simulink library "Renault_Library"  (Simulink 9.2)
settings: Solver=ode3  StartTime=0.0  StopTime=10.0  FixedStep=0.020  RelTol=1e-3  EnableMultiTasking=on
undefined symbols (not defined in the file or in its folder): ABC, AssystemProposal, b, chkval, CoeffA_C, CoeffB_C, CoeffMean_C, ConfTime_C, DiagDelay_C, DividePercent, FailureConfActive_C, FallingEdgeDuration_C, HighLevelThresholdVoltage_C, HighLevelVoltage_C, HighLevelVoltageTunable_C, InitValue_C, InputVoltRangeMax_C, InputVoltRangeMin_C, LowActiveContact_C, LowLevelThresholdVoltage_C, LowLevelVoltage_C, MaxOperatingVoltage_C, MinOperatingVoltage_C, mV2V_conversion, OUT1, OutOfRangeHigh_C, OutOfRangeLow_C, PinSupplySaturationMax, PinSupplySaturationMin, Res1Value_C, Res2Value_C, Res3Value_C, RisingEdgeDuration_C, RSA_CANSignalFailMonitoring, SampleTime_C, service14Table, service19Table, service22Table, service24Table, service28Table, service2ETable, service2FTable, service31Table, service87Table, SizeXStateDID2FTable, SizeXTable14, SizeXTable19, SizeXTable22, SizeXTable24, SizeXTable28, SizeXTable2E, SizeXTable2F, SizeXTable31, SizeXTable87, SizeYStateDID2FTable, SizeYTable14, SizeYTable19, SizeYTable22, SizeYTable24, SizeYTable28 ... (+22)
undefined symbols (not defined in the file): BatteryVoltage_ActivationThreshold_C, FrameStatus_AbsentValue_C, FrameStatus_ConfAbsentValue_C, MemThreshold_C, NAMEOFTHEMODEL_SAMPLE_TIME_CST, NbAbs1_C, NbConfAbs1_C, NbConfPres1_C, NbPres1_C, NOMDUSIGNAL_FaultConfTime_C, NOMDUSIGNAL_FaultRehabTime_C, NOMDUSIGNAL_switchvalue_C, NOMDUSIGNAL_unavailablevalue_C, PeriodFrame1_C, ResetTime_C, WakeUpTime_C
libraries: $bdroot x112, simulink x75
(part 9 of 32)

== system Renault_Library/Complex blocks/Logic & Bit Operations/RSA_CompareToZero ==
  u : Inport
      settings: AttributesFormatString=Type : %<OutDataTypeStr>
  Compare : RelationalOperator  Operator===  OutDataTypeStr=boolean  RndMeth=Nearest  InputSameDT=on
  Constant : Constant  DisableCoverage=on  Value=cons_zero  OutDataTypeStr=Inherit: Inherit via back propagation  SampleTime=-1  PreserveConstantTs=on
  y : Outport
      settings: AttributesFormatString=Type : %<OutDataTypeStr>\nInit :%<InitialOutput>
  wires:
  Constant/1 -> Compare/2
  u/1 -> Compare/1
  Compare/1 -> y/1

== system Renault_Library/Complex blocks/Logic & Bit Operations/RSA_CompareToZero1 ==
  u : Inport
      settings: AttributesFormatString=Type : %<OutDataTypeStr>
  Compare : RelationalOperator  Operator===  OutDataTypeStr=boolean  RndMeth=Nearest  InputSameDT=on
  Constant : Constant  DisableCoverage=on  Value=cons_zero  OutDataTypeStr=Inherit: Inherit via back propagation  SampleTime=-1  PreserveConstantTs=on
  y : Outport
      settings: AttributesFormatString=Type : %<OutDataTypeStr>\nInit :%<InitialOutput>
  wires:
  Constant/1 -> Compare/2
  u/1 -> Compare/1
  Compare/1 -> y/1

== system Renault_Library/Complex blocks/Logic & Bit Operations/RSA_DetectBooleanChange_v01_0 ==
  U : Inport
  Constant1 : Constant  Value=false  OutDataTypeStr=boolean  SampleTime=-1
  Constant2 : Constant  Value=true  OutDataTypeStr=boolean  SampleTime=-1
  Delay_Input1 : UnitDelay  Description=Store in Global RAM  DisableCoverage=on  InitialCondition=false  SampleTime=-1
      settings: AttributesFormatString=IC = %<X0>
  Delay_Input2 : UnitDelay  Description=Store in Global RAM  DisableCoverage=on  InitialCondition=chkini  SampleTime=-1
      settings: AttributesFormatString=IC = %<X0>
  Logical_Operator : Logic  Operator=XOR  OutDataTypeStr=boolean
  Switch : Switch  Criteria=u2 ~= 0  RndMeth=Zero  InputSameDT=on
      settings: AttributesFormatString=Criteria=%<Criteria>
  Y : Outport
  wires:
  Constant1/1 -> Switch/3
  Logical_Operator/1 -> Switch/1
  Switch/1 -> Y/1
  Delay_Input2/1 -> Switch/2
  Constant2/1 -> Delay_Input2/1
  U/1 -> Delay_Input1/1, Logical_Operator/1
  Delay_Input1/1 -> Logical_Operator/2

== system Renault_Library/Complex blocks/Logic & Bit Operations/RSA_DetectChange ==
  note: Edge
  U : Inport
      settings: AttributesFormatString=Type : %<OutDataTypeStr>
  Delay_Input1 : UnitDelay  Description=Store in Global RAM  DisableCoverage=on  InitialCondition=vinit  SampleTime=-1
      settings: AttributesFormatString=IC = %<X0>
  FixPt_Relational_Operator : RelationalOperator  Operator=~=  OutDataTypeStr=boolean  ZeroCross=off  RndMeth=Nearest  InputSameDT=on
  Y : Outport  InitialOutput=0
      settings: AttributesFormatString=Type : %<OutDataTypeStr>\nInit :%<InitialOutput>
  wires:
  FixPt_Relational_Operator/1 -> Y/1
  Delay_Input1/1 -> FixPt_Relational_Operator/2
  U/1 -> FixPt_Relational_Operator/1, Delay_Input1/1

== system Renault_Library/Complex blocks/Logic & Bit Operations/RSA_DetectChange_v01_03 ==
  U : Inport
  Constant1 : Constant  Value=false  OutDataTypeStr=Inherit: Inherit via back propagation  SampleTime=-1
  Constant2 : Constant  Value=false  OutDataTypeStr=boolean  SampleTime=-1
  Delay_Input1 : UnitDelay  Description=Store in Global RAM  DisableCoverage=on  InitialCondition=vinit  SampleTime=-1
      settings: AttributesFormatString=IC = %<X0>
  Delay_Input2 : UnitDelay  Description=Store in Global RAM  DisableCoverage=on  InitialCondition=true  SampleTime=-1
      settings: AttributesFormatString=IC = %<X0>
  FixPt_Relational_Operator : RelationalOperator  Operator=~=  OutDataTypeStr=boolean  ZeroCross=off  RndMeth=Nearest  InputSameDT=on
  Switch : Switch  Criteria=u2 ~= 0  RndMeth=Zero  InputSameDT=on
      settings: AttributesFormatString=Criteria=%<Criteria>
  Y : Outport  InitialOutput=0
  wires:
  Switch/1 -> Y/1  "Edge"
  FixPt_Relational_Operator/1 -> Switch/3
  Constant1/1 -> Switch/1
  Constant2/1 -> Delay_Input2/1
  Delay_Input2/1 -> Switch/2
  Delay_Input1/1 -> FixPt_Relational_Operator/2
  U/1 -> FixPt_Relational_Operator/1, Delay_Input1/1

== system Renault_Library/Complex blocks/Logic & Bit Operations/RSA_DetectChange_v01_04 ==
  U : Inport
  Constant1 : Constant  Value=false  OutDataTypeStr=Inherit: Inherit via back propagation  SampleTime=-1
  Constant2 : Constant  Value=true  OutDataTypeStr=boolean  SampleTime=-1
  Delay_Input1 : UnitDelay  Description=Store in Global RAM  DisableCoverage=on  InitialCondition=vinit  SampleTime=-1
      settings: AttributesFormatString=IC = %<X0>
  Delay_Input2 : UnitDelay  Description=Store in Global RAM  DisableCoverage=on  InitialCondition=chkval  SampleTime=-1
      settings: AttributesFormatString=IC = %<X0>
  FixPt_Relational_Operator : RelationalOperator  Operator=~=  OutDataTypeStr=boolean  ZeroCross=off  RndMeth=Nearest  InputSameDT=on
  Switch : Switch  Criteria=u2 ~= 0  RndMeth=Zero  InputSameDT=on
      settings: AttributesFormatString=Criteria=%<Criteria>
  Y : Outport  InitialOutput=0
  wires:
  U/1 -> Delay_Input1/1, FixPt_Relational_Operator/1
  Delay_Input1/1 -> FixPt_Relational_Operator/2
  Switch/1 -> Y/1  "Edge"
  Constant2/1 -> Delay_Input2/1
  FixPt_Relational_Operator/1 -> Switch/1
  Constant1/1 -> Switch/3
  Delay_Input2/1 -> Switch/2

== system Renault_Library/Complex blocks/Logic & Bit Operations/RSA_DetectChange_v01_05 ==
  U : Inport
  Add : Sum  Inputs=+-  OutDataTypeStr=Inherit: Inherit via back propagation  RndMeth=Zero  InputSameDT=on
      settings: AttributesFormatString=SAT = %<SaturateOnIntegerOverflow>
  Add1 : Sum  OutDataTypeStr=Inherit: Inherit via back propagation  RndMeth=Zero  InputSameDT=on
      settings: AttributesFormatString=SAT = %<SaturateOnIntegerOverflow>
  Con3 : Constant  Value=tol  OutDataTypeStr=Inherit: Inherit via back propagation
  Con5 : Constant  Value=tol  OutDataTypeStr=Inherit: Inherit via back propagation
  Constant2 : Constant  Value=true  OutDataTypeStr=boolean  SampleTime=-1
  Constant3 : Constant  Value=false  OutDataTypeStr=boolean  SampleTime=-1
  Delay_Input1 : UnitDelay  Description=Store in Global RAM  DisableCoverage=on  InitialCondition=vinit  SampleTime=-1
      settings: AttributesFormatString=IC = %<X0>
  Delay_Input2 : UnitDelay  Description=Store in Global RAM  DisableCoverage=on  InitialCondition=false  SampleTime=-1
      settings: AttributesFormatString=IC = %<X0>
  Logical_Operator12 : Logic  Operator=XOR  OutDataTypeStr=boolean
  Switch : Switch  Criteria=u2 ~= 0  RndMeth=Zero  InputSameDT=on
      settings: AttributesFormatString=Criteria=%<Criteria>
  Terminator : Terminator
  Terminator1 : Terminator
  Var_RelationalOper1 : RelationalOperator  Operator=>  OutDataTypeStr=boolean  RndMeth=Nearest  InputSameDT=on
  Var_RelationalOper2 : RelationalOperator  Operator=<  OutDataTypeStr=boolean  RndMeth=Nearest  InputSameDT=on
  Y : Outport  InitialOutput=0
  wires:
  Delay_Input2/1 -> Switch/2
  [fromRelOpe] -> Switch/3
  Constant2/1 -> Delay_Input2/1
  Switch/1 -> Y/1  "Edge"
  Delay_Input1/1 -> [Uprev]
  Constant3/1 -> [vchk_val]
  [compare_out] -> [fromRelOpe], Switch/1
  [vchk_val] -> Terminator/1
  [fromRelOpe] -> Terminator1/1
  Logical_Operator12/1 -> [compare_out]
  Con3/1 -> Add/2
  Con5/1 -> Add1/2
  Add/1 -> Var_RelationalOper1/2
  Var_RelationalOper1/1 -> Logical_Operator12/1
  Var_RelationalOper2/1 -> Logical_Operator12/2
  [U] -> Var_RelationalOper1/1
  [U] -> Var_RelationalOper2/1
  Add1/1 -> Var_RelationalOper2/2
  [Uprev] -> Add/1
  [Uprev] -> Add1/1
  U/1 -> [U], Delay_Input1/1

== system Renault_Library/Complex blocks/Logic & Bit Operations/RSA_DetectDecrease ==
  note: Edge
  U : Inport
      settings: AttributesFormatString=Type : %<OutDataTypeStr>
  Delay_Input1 : UnitDelay  Description=Store in Global RAM  DisableCoverage=on  InitialCondition=vinit  SampleTime=-1
      settings: AttributesFormatString=IC = %<X0>
  FixPt_Relational_Operator : RelationalOperator  Operator=<  OutDataTypeStr=boolean  ZeroCross=off  RndMeth=Nearest  InputSameDT=on
  Y : Outport  InitialOutput=0
      settings: AttributesFormatString=Type : %<OutDataTypeStr>\nInit :%<InitialOutput>
  wires:
  U/1 -> Delay_Input1/1, FixPt_Relational_Operator/1
  Delay_Input1/1 -> FixPt_Relational_Operator/2
  FixPt_Relational_Operator/1 -> Y/1

== system Renault_Library/Complex blocks/Logic & Bit Operations/RSA_DetectDecrease_v01_03 ==
  U : Inport
  Constant1 : Constant  Value=false  OutDataTypeStr=Inherit: Inherit via back propagation  SampleTime=-1
  Constant2 : Constant  Value=false  OutDataTypeStr=boolean  SampleTime=-1
  Delay_Input1 : UnitDelay  Description=Store in Global RAM  DisableCoverage=on  InitialCondition=vinit  SampleTime=-1
      settings: AttributesFormatString=IC = %<X0>
  Delay_Input2 : UnitDelay  Description=Store in Global RAM  DisableCoverage=on  InitialCondition=true  SampleTime=-1
      settings: AttributesFormatString=IC = %<X0>
  FixPt_Relational_Operator : RelationalOperator  Operator=<  OutDataTypeStr=boolean  ZeroCross=off  RndMeth=Nearest  InputSameDT=on
  Switch : Switch  Criteria=u2 ~= 0  RndMeth=Zero  InputSameDT=on
      settings: AttributesFormatString=Criteria=%<Criteria>
  Y : Outport  InitialOutput=0
  wires:
  Delay_Input1/1 -> FixPt_Relational_Operator/2
  U/1 -> FixPt_Relational_Operator/1, Delay_Input1/1
  Delay_Input2/1 -> Switch/2
  Constant2/1 -> Delay_Input2/1
  Constant1/1 -> Switch/1
  FixPt_Relational_Operator/1 -> Switch/3
  Switch/1 -> Y/1  "Edge"

== system Renault_Library/Complex blocks/Logic & Bit Operations/RSA_DetectDecrease_v01_04 ==
  U : Inport
  Constant1 : Constant  Value=false  OutDataTypeStr=Inherit: Inherit via back propagation  SampleTime=-1
  Constant2 : Constant  Value=true  OutDataTypeStr=boolean  SampleTime=-1
  Delay_Input1 : UnitDelay  Description=Store in Global RAM  DisableCoverage=on  InitialCondition=vinit  SampleTime=-1
      settings: AttributesFormatString=IC = %<X0>
  Delay_Input2 : UnitDelay  Description=Store in Global RAM  DisableCoverage=on  InitialCondition=chkval  SampleTime=-1
      settings: AttributesFormatString=IC = %<X0>
  FixPt_Relational_Operator : RelationalOperator  Operator=<  OutDataTypeStr=boolean  ZeroCross=off  RndMeth=Nearest  InputSameDT=on
  Switch : Switch  Criteria=u2 ~= 0  RndMeth=Zero  InputSameDT=on
      settings: AttributesFormatString=Criteria=%<Criteria>
  Y : Outport  InitialOutput=0
  wires:
  Delay_Input1/1 -> FixPt_Relational_Operator/2
  U/1 -> FixPt_Relational_Operator/1, Delay_Input1/1
  Delay_Input2/1 -> Switch/2
  Constant2/1 -> Delay_Input2/1
  Switch/1 -> Y/1  "Edge"
  FixPt_Relational_Operator/1 -> Switch/1
  Constant1/1 -> Switch/3

== system Renault_Library/Complex blocks/Logic & Bit Operations/RSA_DetectIncDec_V01_00 ==
  U : Inport
  Constant2 : Constant  Value=true  OutDataTypeStr=boolean  SampleTime=-1
  Constant3 : Constant  Value=false  OutDataTypeStr=boolean  SampleTime=-1
  Delay_Input1 : UnitDelay  Description=Store in Global RAM  DisableCoverage=on  InitialCondition=vinit  SampleTime=-1
      settings: AttributesFormatString=IC = %<X0>
  Delay_Input2 : UnitDelay  Description=Store in Global RAM  DisableCoverage=on  InitialCondition=false  SampleTime=-1
      settings: AttributesFormatString=IC = %<X0>
  FixPt_Relational_Operator : RelationalOperator  Operator=<  OutDataTypeStr=boolean  ZeroCross=off  RndMeth=Nearest  InputSameDT=on
  Switch : Switch  Criteria=u2 ~= 0  RndMeth=Zero  InputSameDT=on
      settings: AttributesFormatString=Criteria=%<Criteria>
  Terminator : Terminator
  Terminator1 : Terminator
  Y : Outport  InitialOutput=0
  wires:
  Switch/1 -> Y/1  "Edge"
  [fromRelOpe] -> Terminator1/1
  [vchk_val] -> Terminator/1
  FixPt_Relational_Operator/1 -> Switch/1, [fromRelOpe]
  [vchk_val] -> Switch/3
  Constant2/1 -> Delay_Input2/1
  Constant3/1 -> [vchk_val]
  Delay_Input2/1 -> Switch/2
  U/1 -> Delay_Input1/1, FixPt_Relational_Operator/1
  Delay_Input1/1 -> FixPt_Relational_Operator/2

== system Renault_Library/Complex blocks/Logic & Bit Operations/RSA_DetectIncrease ==
  note: Edge
  U : Inport
      settings: AttributesFormatString=Type : %<OutDataTypeStr>
  Delay_Input1 : UnitDelay  Description=Store in Global RAM  DisableCoverage=on  InitialCondition=vinit  SampleTime=-1
      settings: AttributesFormatString=IC = %<X0>
  FixPt_Relational_Operator : RelationalOperator  Operator=>  OutDataTypeStr=boolean  ZeroCross=off  RndMeth=Nearest  InputSameDT=on
  Y : Outport  InitialOutput=0
      settings: AttributesFormatString=Type : %<OutDataTypeStr>\nInit :%<InitialOutput>
  wires:
  U/1 -> Delay_Input1/1, FixPt_Relational_Operator/1
  Delay_Input1/1 -> FixPt_Relational_Operator/2
  FixPt_Relational_Operator/1 -> Y/1

== system Renault_Library/Complex blocks/Logic & Bit Operations/RSA_DetectIncrease_v01_03 ==
  U : Inport
  Constant1 : Constant  Value=false  OutDataTypeStr=Inherit: Inherit via back propagation  SampleTime=-1
  Constant2 : Constant  Value=false  OutDataTypeStr=boolean  SampleTime=-1
  Delay_Input1 : UnitDelay  Description=Store in Global RAM  DisableCoverage=on  InitialCondition=vinit  SampleTime=-1
      settings: AttributesFormatString=IC = %<X0>
  Delay_Input2 : UnitDelay  Description=Store in Global RAM  DisableCoverage=on  InitialCondition=true  SampleTime=-1
      settings: AttributesFormatString=IC = %<X0>
  FixPt_Relational_Operator : RelationalOperator  Operator=>  OutDataTypeStr=boolean  ZeroCross=off  RndMeth=Nearest  InputSameDT=on
  Switch : Switch  Criteria=u2 ~= 0  RndMeth=Zero  InputSameDT=on
      settings: AttributesFormatString=Criteria=%<Criteria>
  Y : Outport  InitialOutput=0
  wires:
  Delay_Input1/1 -> FixPt_Relational_Operator/2
  U/1 -> FixPt_Relational_Operator/1, Delay_Input1/1
  Delay_Input2/1 -> Switch/2
  Constant2/1 -> Delay_Input2/1
  Constant1/1 -> Switch/1
  Switch/1 -> Y/1  "Edge"
  FixPt_Relational_Operator/1 -> Switch/3

== system Renault_Library/Complex blocks/Logic & Bit Operations/RSA_DetectIncrease_v01_04 ==
  U : Inport
  Constant1 : Constant  Value=false  OutDataTypeStr=Inherit: Inherit via back propagation  SampleTime=-1
  Constant2 : Constant  Value=true  OutDataTypeStr=boolean  SampleTime=-1
  Delay_Input1 : UnitDelay  Description=Store in Global RAM  DisableCoverage=on  InitialCondition=vinit  SampleTime=-1
      settings: AttributesFormatString=IC = %<X0>
  Delay_Input2 : UnitDelay  Description=Store in Global RAM  DisableCoverage=on  InitialCondition=chkval  SampleTime=-1
      settings: AttributesFormatString=IC = %<X0>
  FixPt_Relational_Operator : RelationalOperator  Operator=>  OutDataTypeStr=boolean  ZeroCross=off  RndMeth=Nearest  InputSameDT=on
  Switch : Switch  Criteria=u2 ~= 0  RndMeth=Zero  InputSameDT=on
      settings: AttributesFormatString=Criteria=%<Criteria>
  Y : Outport  InitialOutput=0
  wires:
  Switch/1 -> Y/1  "Edge"
  Constant2/1 -> Delay_Input2/1
  Delay_Input2/1 -> Switch/2
  U/1 -> Delay_Input1/1, FixPt_Relational_Operator/1
  Delay_Input1/1 -> FixPt_Relational_Operator/2
  FixPt_Relational_Operator/1 -> Switch/1
  Constant1/1 -> Switch/3

== system Renault_Library/Complex blocks/Logic & Bit Operations/RSA_EdgeDetect ==
  In : Inport
      settings: AttributesFormatString=Type : %<OutDataType>\nScaling : %<OutScaling>
  IC : Inport  Port=2
      settings: AttributesFormatString=Type : %<OutDataType>\nScaling : %<OutScaling>
  Edge_type : Constant  Value=Edge_type  OutDataTypeStr=uint8  SampleTime=-1
  Logical_Operator : Logic  OutDataTypeStr=boolean
  Logical_Operator1 : Logic  Operator=NOT  OutDataTypeStr=boolean
  Logical_Operator2 : Logic  OutDataTypeStr=boolean
  Logical_Operator3 : Logic  Operator=NOT  OutDataTypeStr=boolean
  Logical_Operator4 : Logic  Operator=XOR  OutDataTypeStr=boolean
  Multiport_Switch : MultiPortSwitch  RndMeth=Zero  SaturateOnIntegerOverflow=on  InputSameDT=on
  RSA_UnitDelayExternalIC_v01_03 : $bdroot/Complex blocks/Discrete/RSA_UnitDelayExternalIC_v01_03  Tag=[LIB]  SystemSampleTime=-1
      settings: AttributesFormatString=%<MaskType> : Ver : 01.03
  Out : Outport
      settings: AttributesFormatString=Type : %<OutDataType>\nScaling : %<OutScaling>\nInit :%<InitialOutput>
  wires:
  In/1 -> Logical_Operator/1, Logical_Operator3/1, Logical_Operator4/1, RSA_UnitDelayExternalIC_v01_03/1
  Logical_Operator1/1 -> Logical_Operator/2
  Logical_Operator3/1 -> Logical_Operator2/1
  Logical_Operator/1 -> Multiport_Switch/2  "Rising"
  Logical_Operator2/1 -> Multiport_Switch/3  "Falling"
  Logical_Operator4/1 -> Multiport_Switch/4  "Either"
  Edge_type/1 -> Multiport_Switch/1
  Multiport_Switch/1 -> Out/1
  IC/1 -> RSA_UnitDelayExternalIC_v01_03/2
  RSA_UnitDelayExternalIC_v01_03/1 -> [y]
  [y] -> Logical_Operator1/1
  [y] -> Logical_Operator2/2
  [y] -> Logical_Operator4/2

== system Renault_Library/Complex blocks/Logic & Bit Operations/RSA_EdgeDetect_V01_02 ==
  In : Inport
      settings: AttributesFormatString=Type : %<OutDataType>\nScaling : %<OutScaling>
  IC : Inport  Port=2
      settings: AttributesFormatString=Type : %<OutDataType>\nScaling : %<OutScaling>
  Logical_Operator : Logic  OutDataTypeStr=boolean
  Logical_Operator1 : Logic  Operator=NOT  OutDataTypeStr=boolean
  Logical_Operator2 : Logic  OutDataTypeStr=boolean
  Logical_Operator3 : Logic  Operator=NOT  OutDataTypeStr=boolean
  Logical_Operator4 : Logic  Operator=XOR  OutDataTypeStr=boolean
  RSA_UnitDelayExternalIC_v01_03 : $bdroot/Complex blocks/Discrete/RSA_UnitDelayExternalIC_v01_03  Tag=[LIB]  SystemSampleTime=-1
      settings: AttributesFormatString=%<MaskType> : Ver : 01.03
  Terminator : Terminator
  Terminator1 : Terminator
  Terminator2 : Terminator
  Out : Outport
      settings: AttributesFormatString=Type : %<OutDataType>\nScaling : %<OutScaling>\nInit :%<InitialOutput>
  wires:
  In/1 -> Logical_Operator/1, Logical_Operator3/1, Logical_Operator4/1, RSA_UnitDelayExternalIC_v01_03/1
  Logical_Operator1/1 -> Logical_Operator/2
  Logical_Operator3/1 -> Logical_Operator2/1
  IC/1 -> RSA_UnitDelayExternalIC_v01_03/2
  RSA_UnitDelayExternalIC_v01_03/1 -> [y]
  [y] -> Logical_Operator1/1
  [y] -> Logical_Operator2/2
  [y] -> Logical_Operator4/2
  [FallingEdge] -> Out/1
  Logical_Operator/1 -> [RisingEdge]  "Rising"
  Logical_Operator2/1 -> [FallingEdge]  "Falling"
  Logical_Operator4/1 -> [Either]  "Either"
  [RisingEdge] -> Terminator/1
  [FallingEdge] -> Terminator1/1
  [Either] -> Terminator2/1

== system Renault_Library/Complex blocks/Lookup Tables/RSA_Cosine_v01_03 ==
  u : Inport
      settings: AttributesFormatString=Type : %<OutDataTypeStr>
  Amp25 : Sum  Inputs=+-  OutDataTypeStr=Inherit: Inherit via back propagation  InputSameDT=on  LockScale=on
      settings: AttributesFormatString=SAT = %<SaturateOnIntegerOverflow>
  Amp75 : Sum  Inputs=+-  OutDataTypeStr=Inherit: Inherit via back propagation  InputSameDT=on  LockScale=on
      settings: AttributesFormatString=SAT = %<SaturateOnIntegerOverflow>
  Constant11 : Constant  Value=NVAL  OutDataTypeStr=Inherit: Inherit via back propagation  SampleTime=-1
  DataTypeConversion : DataTypeConversion  OutDataTypeStr=fixdt(0, OutputWordLength, OutputWordLength)
  GTEp75 : RelationalOperator  OutDataTypeStr=boolean  ZeroCross=off  RndMeth=Nearest  InputSameDT=on
  LTEp25 : RelationalOperator  Operator=<=  OutDataTypeStr=boolean  ZeroCross=off  RndMeth=Nearest  InputSameDT=on
  LTEp50 : RelationalOperator  Operator=<=  OutDataTypeStr=boolean  ZeroCross=off  RndMeth=Nearest  InputSameDT=on
  Look_Up_Table : Lookup  InputValues=k  Table=b  LookUpMeth=Interpolation-Use End Values  OutDataTypeStr=fixdt(1, OutputWordLength, OutputFracLength)  RndMeth=Zero  SaturateOnIntegerOverflow=on
  Point25 : Constant  Value=0.25  OutDataTypeStr=fixdt(0, OutputWordLength, OutputWordLength)
  Point5 : Constant  Value=0.5  OutDataTypeStr=fixdt(0, OutputWordLength, OutputWordLength)
  Point75 : Constant  Value=0.75  OutDataTypeStr=fixdt(0, OutputWordLength, OutputWordLength)
  Product2 : Product  OutDataTypeStr=Inherit: Inherit via back propagation  RndMeth=Zero
      settings: AttributesFormatString=SAT = %<SaturateOnIntegerOverflow>
  QuadHandle1a : Switch  Criteria=u2 ~= 0  ZeroCross=off  InputSameDT=on
      settings: AttributesFormatString=Criteria=%<Criteria>
  QuadHandle1b : Switch  Criteria=u2 ~= 0  ZeroCross=off  InputSameDT=on
      settings: AttributesFormatString=Criteria=%<Criteria>
  QuadHandle2 : Switch  Criteria=u2 ~= 0  ZeroCross=off  InputSameDT=on
      settings: AttributesFormatString=Criteria=%<Criteria>
  SignCorrected : Switch  Criteria=u2 ~= 0  ZeroCross=off  InputSameDT=on
      settings: AttributesFormatString=Criteria=%<Criteria>
  firstor4thQuad : Logic  Operator=OR  OutDataTypeStr=boolean
  p25mA : Sum  Inputs=+-  OutDataTypeStr=Inherit: Inherit via back propagation  InputSameDT=on  LockScale=on
      settings: AttributesFormatString=SAT = %<SaturateOnIntegerOverflow>
  p75mA : Sum  Inputs=+-  OutDataTypeStr=Inherit: Inherit via back propagation  InputSameDT=on  LockScale=on
      settings: AttributesFormatString=SAT = %<SaturateOnIntegerOverflow>
  y : Outport
      settings: AttributesFormatString=Type : %<OutDataTypeStr>\nInit :%<InitialOutput>
  wires:
  QuadHandle2/1 -> Look_Up_Table/1
  Constant11/1 -> Product2/2
  SignCorrected/1 -> y/1
  Product2/1 -> SignCorrected/3
  Look_Up_Table/1 -> Product2/1, SignCorrected/1
  [fourthquad] -> firstor4thQuad/2
  [firstquad] -> firstor4thQuad/1
  firstor4thQuad/1 -> SignCorrected/2
  [Point75] -> p75mA/1
  QuadHandle1b/1 -> QuadHandle2/3
  QuadHandle1a/1 -> QuadHandle2/1
  LTEp50/1 -> QuadHandle2/2
  Point5/1 -> LTEp50/2
  p75mA/1 -> QuadHandle1b/3
  [Point75] -> Amp75/2
  [Point75] -> GTEp75/2
  Point75/1 -> [Point75]
  Amp75/1 -> QuadHandle1b/1
  GTEp75/1 -> [fourthquad], QuadHandle1b/2
  [Point25] -> p25mA/1
  [Point25] -> Amp25/2
  [Point25] -> LTEp25/2
  Point25/1 -> [Point25]
  Amp25/1 -> QuadHandle1a/3
  p25mA/1 -> QuadHandle1a/1
  LTEp25/1 -> [firstquad], QuadHandle1a/2
  DataTypeConversion/1 -> LTEp50/1, p75mA/2, GTEp75/1, Amp75/1, Amp25/1, p25mA/2, LTEp25/1
  u/1 -> DataTypeConversion/1

== system Renault_Library/Complex blocks/Lookup Tables/RSA_Cosine_v01_04 ==
  u : Inport
      settings: AttributesFormatString=Type : %<OutDataTypeStr>
  Amp25 : Sum  Inputs=+-  OutDataTypeStr=Inherit: Inherit via back propagation  RndMeth=Zero  InputSameDT=on  LockScale=on
      settings: AttributesFormatString=SAT = %<SaturateOnIntegerOverflow>
  Amp75 : Sum  Inputs=+-  OutDataTypeStr=Inherit: Inherit via back propagation  RndMeth=Zero  InputSameDT=on  LockScale=on
      settings: AttributesFormatString=SAT = %<SaturateOnIntegerOverflow>
  Constant11 : Constant  Value=CONS_M1  OutDataTypeStr=Inherit: Inherit via back propagation  SampleTime=-1
  DataTypeConversion : DataTypeConversion  OutDataTypeStr=fixdt(0, OutputWordLength, OutputWordLength)  RndMeth=Zero
  GTEp75 : RelationalOperator  OutDataTypeStr=boolean  ZeroCross=off  RndMeth=Nearest  InputSameDT=on
  LTEp25 : RelationalOperator  Operator=<=  OutDataTypeStr=boolean  ZeroCross=off  RndMeth=Nearest  InputSameDT=on
  LTEp50 : RelationalOperator  Operator=<=  OutDataTypeStr=boolean  ZeroCross=off  RndMeth=Nearest  InputSameDT=on
  Look_Up_Table : Lookup  InputValues=k  Table=b  LookUpMeth=Interpolation-Use End Values  OutDataTypeStr=fixdt(1, OutputWordLength, OutputFracLength)  RndMeth=Zero  SaturateOnIntegerOverflow=on
  Point25 : Constant  Value=CONS_P25  OutDataTypeStr=fixdt(0, OutputWordLength, OutputWordLength)
  Point5 : Constant  Value=CONS_P5  OutDataTypeStr=fixdt(0, OutputWordLength, OutputWordLength)
  Point75 : Constant  Value=CONS_P75  OutDataTypeStr=fixdt(0, OutputWordLength, OutputWordLength)
  Product2 : Product  OutDataTypeStr=Inherit: Inherit via back propagation  RndMeth=Zero
      settings: AttributesFormatString=SAT = %<SaturateOnIntegerOverflow>
  QuadHandle1a : Switch  Criteria=u2 ~= 0  RndMeth=Zero  ZeroCross=off  InputSameDT=on
      settings: AttributesFormatString=Criteria=%<Criteria>
  QuadHandle1b : Switch  Criteria=u2 ~= 0  RndMeth=Zero  ZeroCross=off  InputSameDT=on
      settings: AttributesFormatString=Criteria=%<Criteria>
  QuadHandle2 : Switch  Criteria=u2 ~= 0  RndMeth=Zero  ZeroCross=off  InputSameDT=on
      settings: AttributesFormatString=Criteria=%<Criteria>
  SignCorrected : Switch  Criteria=u2 ~= 0  RndMeth=Zero  ZeroCross=off  InputSameDT=on
      settings: AttributesFormatString=Criteria=%<Criteria>
  firstor4thQuad : Logic  Operator=OR  OutDataTypeStr=boolean
  p25mA : Sum  Inputs=+-  OutDataTypeStr=Inherit: Inherit via back propagation  RndMeth=Zero  InputSameDT=on  LockScale=on
      settings: AttributesFormatString=SAT = %<SaturateOnIntegerOverflow>
  p75mA : Sum  Inputs=+-  OutDataTypeStr=Inherit: Inherit via back propagation  RndMeth=Zero  InputSameDT=on  LockScale=on
      settings: AttributesFormatString=SAT = %<SaturateOnIntegerOverflow>
  y : Outport
      settings: AttributesFormatString=Type : %<OutDataTypeStr>\nInit :%<InitialOutput>
  wires:
  QuadHandle2/1 -> Look_Up_Table/1
  Constant11/1 -> Product2/2
  SignCorrected/1 -> y/1
  Product2/1 -> SignCorrected/3
  Look_Up_Table/1 -> Product2/1, SignCorrected/1
  [fourthquad] -> firstor4thQuad/2
  [firstquad] -> firstor4thQuad/1
  firstor4thQuad/1 -> SignCorrected/2
  [Point75] -> p75mA/1
  QuadHandle1b/1 -> QuadHandle2/3
  QuadHandle1a/1 -> QuadHandle2/1
  LTEp50/1 -> QuadHandle2/2
  Point5/1 -> LTEp50/2
  p75mA/1 -> QuadHandle1b/3
  [Point75] -> Amp75/2
  [Point75] -> GTEp75/2
  Point75/1 -> [Point75]
  Amp75/1 -> QuadHandle1b/1
  GTEp75/1 -> [fourthquad], QuadHandle1b/2
  [Point25] -> p25mA/1
  [Point25] -> Amp25/2
  [Point25] -> LTEp25/2
  Point25/1 -> [Point25]
  Amp25/1 -> QuadHandle1a/3
  p25mA/1 -> QuadHandle1a/1
  LTEp25/1 -> [firstquad], QuadHandle1a/2
  DataTypeConversion/1 -> LTEp50/1, p75mA/2, GTEp75/1, Amp75/1, Amp25/1, p25mA/2, LTEp25/1
  u/1 -> DataTypeConversion/1

== system Renault_Library/Complex blocks/Lookup Tables/RSA_LookupTableDynamic_v02_00 ==
  x : Inport
  xdat : Inport  Port=2
  ydat : Inport  Port=3
  Constant4 : Constant  Value=cons1  OutDataTypeStr=uint8  SampleTime=-1
  DataTypeConversion1 : DataTypeConversion  OutDataTypeStr=uint8  RndMeth=Zero
  DataTypeConversion1_16 : DataTypeConversion  OutDataTypeStr=uint8  RndMeth=Zero
  Demux : Demux  Outputs=2
  Demux1 : Demux  Outputs=2
  Divide : Product  Tag=[LIB]  Inputs=*/  OutDataTypeStr=datt  SaturateOnIntegerOverflow=on  RndMeth=Zero
      settings: AttributesFormatString=SAT = %<SaturateOnIntegerOverflow>
  MinMax : MinMax  OutDataTypeStr=Inherit: Inherit via back propagation  RndMeth=Zero  InputSameDT=on
  MinMax1 : MinMax  Function=max  OutDataTypeStr=Inherit: Inherit via back propagation  RndMeth=Zero  InputSameDT=on
  Mux2 : Mux  Inputs=2
  Mux3 : Mux  Inputs=2
  Mux4 : Mux  Inputs=2
  Product1 : Product  OutDataTypeStr=Inherit: Inherit via back propagation  RndMeth=Nearest  SaturateOnIntegerOverflow=on
      settings: AttributesFormatString=SAT = %<SaturateOnIntegerOverflow>
  Product2 : Product  OutDataTypeStr=Inherit: Inherit via back propagation  RndMeth=Nearest  SaturateOnIntegerOverflow=on
      settings: AttributesFormatString=SAT = %<SaturateOnIntegerOverflow>
  RelationalOperator : RelationalOperator  Tag=AM,Std,2,1;CMP,Operator;  Operator=~=  OutDataTypeStr=boolean  RndMeth=Nearest  InputSameDT=on
  RelationalOperator1 : RelationalOperator  OutDataTypeStr=boolean  RndMeth=Nearest  InputSameDT=on
  RelationalOperator2 : RelationalOperator  Operator=<=  OutDataTypeStr=boolean  RndMeth=Nearest  InputSameDT=on
  RelationalOperator3 : RelationalOperator  OutDataTypeStr=boolean  RndMeth=Nearest  InputSameDT=on
  Relational_Operator : RelationalOperator  Operator=<=  OutDataTypeStr=boolean  RndMeth=Nearest  InputSameDT=on
  Relational_Operator1 : RelationalOperator  OutDataTypeStr=boolean  RndMeth=Nearest  InputSameDT=on
  Relational_Operator2 : RelationalOperator  Operator===  OutDataTypeStr=boolean  RndMeth=Nearest  InputSameDT=on
  Relational_Operator3 : RelationalOperator  Operator=>  OutDataTypeStr=boolean  RndMeth=Nearest  InputSameDT=on
  Selector{1,3..5} (4 alike) : Selector  IndexOptions=Index vector (port)  Indices=SelIdx  OutputSizes=1
  Sum1 : Sum  Inputs=+-  OutDataTypeStr=Inherit: Inherit via back propagation  RndMeth=Zero  SaturateOnIntegerOverflow=on  InputSameDT=on
      settings: AttributesFormatString=SAT = %<SaturateOnIntegerOverflow>
  Sum{2,6..8} (4 alike) : Sum  Inputs=-+  OutDataTypeStr=Inherit: Inherit via back propagation  RndMeth=Zero  SaturateOnIntegerOverflow=on  InputSameDT=on
      settings: AttributesFormatString=SAT = %<SaturateOnIntegerOverflow>
  Sum3 : Sum  OutDataTypeStr=Inherit: Inherit via back propagation  RndMeth=Zero  SaturateOnIntegerOverflow=on  InputSameDT=on
      settings: AttributesFormatString=SAT = %<SaturateOnIntegerOverflow>
  Sum4 : Sum  OutDataTypeStr=Inherit: Inherit via back propagation  RndMeth=Zero  SaturateOnIntegerOverflow=on  InputSameDT=on
      settings: AttributesFormatString=SAT = %<SaturateOnIntegerOverflow>
  Sum_of_Elements : Sum  Inputs=+  OutDataTypeStr=Inherit: Inherit via back propagation  RndMeth=Zero  InputSameDT=on
      settings: AttributesFormatString=SAT = %<SaturateOnIntegerOverflow>
  SumofElements2 : Sum  Inputs=+  OutDataTypeStr=Inherit: Inherit via back propagation  RndMeth=Zero  InputSameDT=on
      settings: AttributesFormatString=SAT = %<SaturateOnIntegerOverflow>
  Switch{,1..4} (5 alike) : Switch  Criteria=u2 ~= 0  RndMeth=Zero  InputSameDT=on
      settings: AttributesFormatString=Criteria=%<Criteria>
  Switch5 : Switch  Tag=GEN,Threshold;AM,Switch,2,1;CMP,Threshold;  Criteria=u2 ~= 0  RndMeth=Zero  SaturateOnIntegerOverflow=on  InputSameDT=on
      settings: AttributesFormatString=Criteria=%<Criteria>
  imm_zero : Constant  Tag=AM,Constant,0,1,Value;CMP,Value;  Value=divchk  OutDataTypeStr=Inherit: Inherit via back propagation  SampleTime=-1
  tempx1 : Selector  IndexOptions=Index vector (port)  Indices=SelIdx  OutputSizes=2
  tempx2 : Selector  IndexOptions=Index vector (port)  Indices=SelIdx  OutputSizes=2
  y : Outport
  wires:
  [temp2] -> tempx2/2
  [cons1] -> Mux2/1
  Switch2/1 -> [temp2], tempx1/2
  [cons1] -> Mux2/2
  Switch4/1 -> Switch2/3
  Mux4/1 -> Switch4/1
  [ylen] -> Mux4/2
  [ylen] -> Mux4/1
  RelationalOperator3/1 -> Switch4/2
  [ylen] -> Selector3/2
  Selector3/1 -> RelationalOperator3/2
  [x] -> RelationalOperator3/1
  [xdat] -> Selector3/1
  Mux2/1 -> Switch2/1
  Mux3/1 -> Switch4/3
  [xdat] -> RelationalOperator1/2
  [x] -> RelationalOperator1/1
  RelationalOperator2/1 -> Switch2/2
  Selector1/1 -> RelationalOperator2/2
  [x] -> RelationalOperator2/1
  [xdat] -> Selector1/1
  [cons1] -> Selector1/2
  SumofElements2/1 -> Mux3/1, Sum4/1
  [cons1] -> Sum4/2
  Sum4/1 -> Mux3/2
  DataTypeConversion1/1 -> SumofElements2/1
  RelationalOperator1/1 -> DataTypeConversion1/1
  Switch1/1 -> Switch/3
  Relational_Operator1/1 -> Switch1/2
  [x] -> Relational_Operator1/1
  Relational_Operator/1 -> Switch/2
  [x] -> Relational_Operator/1
  [xdat] -> MinMax/1
  [xdat] -> MinMax1/1
  MinMax1/1 -> Relational_Operator1/2
  MinMax/1 -> Relational_Operator/2
  Selector4/1 -> Switch/1
  [ydat] -> Selector4/1
  Selector5/1 -> Switch1/1
  [ydat] -> Selector5/1, Relational_Operator2/1, Relational_Operator2/2
  DataTypeConversion1_16/1 -> Sum_of_Elements/1
  Relational_Operator2/1 -> DataTypeConversion1_16/1
  Sum_of_Elements/1 -> [ylen], Selector5/2
  Demux/1 -> Sum1/2, Sum6/1
  xdat/1 -> tempx1/1, [xdat]
  Demux/2 -> Sum6/2
  x/1 -> Sum1/1, [x]
  ydat/1 -> [ydat], tempx2/1
  tempx2/1 -> Demux1/1
  Switch/1 -> y/1
  tempx1/1 -> Demux/1
  [cons1] -> Selector4/2
  Constant4/1 -> [cons1]
  [ydat2] -> Sum7/1
  [ydat1] -> Sum7/2
  [ydat1] -> Sum2/1
  [ydat2] -> Sum2/2
  [ydat1] -> Sum8/2
  Sum8/1 -> Switch3/1
  Sum3/1 -> Switch3/3
  [ydat2] -> Relational_Operator3/2
  [ydat1] -> Relational_Operator3/1
  [ydat1] -> Sum3/1
  Product1/1 -> Sum8/1  "y0"
  Sum7/1 -> Product1/1
  Product2/1 -> Sum3/2
  Sum2/1 -> Product2/2
  Relational_Operator3/1 -> Switch3/2
  Demux1/1 -> [ydat1]
  Demux1/2 -> [ydat2]
  Switch3/1 -> Switch1/3
  Switch5/1 -> Product2/1, Product1/2
  Sum1/1 -> [nume]
  Sum6/1 -> [dnom]
  imm_zero/1 -> RelationalOperator/2
  Divide/1 -> Switch5/1
  RelationalOperator/1 -> Switch5/2
  [dnom] -> RelationalOperator/1
  [nume] -> Divide/1
  [dnom] -> Divide/2
  [nume] -> Switch5/3

== system Renault_Library/Complex blocks/Lookup Tables/RSA_LookupTableDynamic_v02_01 ==
  note: %% This implmentation is to avoid different signal dimensions on XDAT and YDAT.
      | %% If Different dimension is given to the block, this part will throw a simulation error.
  x : Inport
  xdat : Inport  Port=2
  ydat : Inport  Port=3
  Constant1 : Constant  Value=cons1  OutDataTypeStr=uint8  SampleTime=-1
  Constant2 : Constant  Value=false  OutDataTypeStr=boolean  SampleTime=-1
  Constant4 : Constant  Value=cons0  OutDataTypeStr=uint8  SampleTime=-1
  Data Type Conversion : DataTypeConversion
  DataTypeConversion1 : DataTypeConversion  OutDataTypeStr=uint8  RndMeth=Zero
  DataTypeConversion1_2 : DataTypeConversion  OutDataTypeStr=uint8  RndMeth=Zero
  DataTypeConversion1_3 : DataTypeConversion  OutDataTypeStr=uint8  RndMeth=Zero
  Demux : Demux  Outputs=2
  Demux1 : Demux  Outputs=2
  Divide : Product  Tag=[LIB]  Inputs=*/  OutDataTypeStr=datt  SaturateOnIntegerOverflow=on  RndMeth=Zero
      settings: AttributesFormatString=SAT = %<SaturateOnIntegerOverflow>
  Mux3 : Mux  Inputs=2
  Product1 : Product  OutDataTypeStr=Inherit: Inherit via back propagation  RndMeth=Nearest  SaturateOnIntegerOverflow=on
      settings: AttributesFormatString=SAT = %<SaturateOnIntegerOverflow>
  Product2 : Product  OutDataTypeStr=Inherit: Inherit via back propagation  RndMeth=Nearest  SaturateOnIntegerOverflow=on
      settings: AttributesFormatString=SAT = %<SaturateOnIntegerOverflow>
  RelationalOperator : RelationalOperator  Tag=AM,Std,2,1;CMP,Operator;  Operator=>  OutDataTypeStr=boolean  RndMeth=Nearest  InputSameDT=on
  RelationalOperator1 : RelationalOperator  OutDataTypeStr=boolean  RndMeth=Nearest  InputSameDT=on
  RelationalOperator2 : RelationalOperator  Tag=AM,Std,2,1;CMP,Operator;  Operator=>  OutDataTypeStr=boolean  RndMeth=Nearest  InputSameDT=on
  Relational_Operator : RelationalOperator  Operator=<=  OutDataTypeStr=boolean  RndMeth=Nearest  InputSameDT=on
  Relational_Operator1 : RelationalOperator  OutDataTypeStr=boolean  RndMeth=Nearest  InputSameDT=on
  Relational_Operator3 : RelationalOperator  Operator===  OutDataTypeStr=boolean  RndMeth=Nearest  InputSameDT=on
  Sat_blk : Subsystem
  Selector{2,4..6} (4 alike) : Selector  IndexMode=Zero-based  IndexOptions=Index vector (port)  Indices=SelIdx  OutputSizes=1
  Sum{1,5,8..9} (4 alike) : Sum  Inputs=+-  OutDataTypeStr=Inherit: Inherit via back propagation  RndMeth=Zero  InputSameDT=on
      settings: AttributesFormatString=SAT = %<SaturateOnIntegerOverflow>
  Sum2 : Sum  Inputs=-+  OutDataTypeStr=Inherit: Inherit via back propagation  RndMeth=Zero  InputSameDT=on
      settings: AttributesFormatString=SAT = %<SaturateOnIntegerOverflow>
  Sum3 : Sum  OutDataTypeStr=Inherit: Inherit via back propagation  RndMeth=Zero  SaturateOnIntegerOverflow=on  InputSameDT=on
      settings: AttributesFormatString=SAT = %<SaturateOnIntegerOverflow>
  Sum4 : Sum  OutDataTypeStr=Inherit: Inherit via back propagation  RndMeth=Zero  InputSameDT=on
      settings: AttributesFormatString=SAT = %<SaturateOnIntegerOverflow>
  Sum6 : Sum  Inputs=-+  OutDataTypeStr=Inherit: Inherit via back propagation  RndMeth=Zero  InputSameDT=on
      settings: AttributesFormatString=SAT = %<SaturateOnIntegerOverflow>
  Sum7 : Sum  Inputs=+-  OutDataTypeStr=Inherit: Inherit via back propagation  RndMeth=Zero  SaturateOnIntegerOverflow=on  InputSameDT=on
      settings: AttributesFormatString=SAT = %<SaturateOnIntegerOverflow>
  Sum_of_Elements1 : Sum  Inputs=+  OutDataTypeStr=Inherit: Inherit via back propagation  RndMeth=Zero  InputSameDT=on
      settings: AttributesFormatString=SAT = %<SaturateOnIntegerOverflow>
  SumofElements2 : Sum  Inputs=+  OutDataTypeStr=Inherit: Inherit via back propagation  RndMeth=Zero  InputSameDT=on
      settings: AttributesFormatString=SAT = %<SaturateOnIntegerOverflow>
  Switch : Switch  Criteria=u2 ~= 0  RndMeth=Zero  InputSameDT=on
      settings: AttributesFormatString=Criteria=%<Criteria>
  Switch1 : Switch  Criteria=u2 ~= 0  RndMeth=Zero  InputSameDT=on
      settings: AttributesFormatString=Criteria=%<Criteria>
  Switch2 : Switch  Criteria=u2 ~= 0  RndMeth=Zero
      settings: AttributesFormatString=Criteria=%<Criteria>
  Switch3 : Switch  Tag=GEN,Threshold;AM,Switch,2,1;CMP,Threshold;  Criteria=u2 ~= 0  RndMeth=Zero  SaturateOnIntegerOverflow=on  InputSameDT=on
      settings: AttributesFormatString=Criteria=%<Criteria>
  Switch5 : Switch  Tag=GEN,Threshold;AM,Switch,2,1;CMP,Threshold;  Criteria=u2 ~= 0  RndMeth=Zero  SaturateOnIntegerOverflow=on  InputSameDT=on
      settings: AttributesFormatString=Criteria=%<Criteria>
  Terminator : Terminator
  UnitDelay : UnitDelay  InitialCondition=true  SampleTime=-1
      settings: AttributesFormatString=IC = %<X0>
  imm_zero : Constant  Tag=AM,Constant,0,1,Value;CMP,Value;  Value=divchk  OutDataTypeStr=Inherit: Inherit via back propagation  SampleTime=-1
  tempx1 : Selector  IndexMode=Zero-based  IndexOptions=Index vector (port)  Indices=SelIdx  OutputSizes=2
  tempx2 : Selector  IndexMode=Zero-based  IndexOptions=Index vector (port)  Indices=SelIdx  OutputSizes=2
  y : Outport
  wires:
  Data Type Conversion/1 -> [nume]
  [temp2] -> tempx2/2
  [xdat] -> RelationalOperator1/2
  [x] -> RelationalOperator1/1
  [cons1] -> Sum9/2, Sum4/2
  DataTypeConversion1/1 -> SumofElements2/1
  RelationalOperator1/1 -> DataTypeConversion1/1
  Switch1/1 -> Switch/3
  Relational_Operator1/1 -> Switch1/2
  [x] -> Relational_Operator1/1
  [x] -> Relational_Operator/1
  Selector6/1 -> Relational_Operator1/2
  Selector4/1 -> Switch/1
  [ydat] -> Selector4/1
  Selector5/1 -> Switch1/1
  Demux/1 -> Sum1/2, Sum6/1
  xdat/1 -> [xdat], tempx1/1
  Demux/2 -> Sum6/2
  x/1 -> Sum1/1, [x]
  ydat/1 -> [ydat], tempx2/1
  tempx2/1 -> Demux1/1
  Switch/1 -> y/1
  tempx1/1 -> Demux/1
  [cons0] -> Selector4/2
  Constant4/1 -> [cons0]
  [ydat1] -> Sum3/1
  Product2/1 -> Sum3/2
  Demux1/1 -> [ydat1]
  Demux1/2 -> [ydat2]
  Sum1/1 -> Data Type Conversion/1
  Sum6/1 -> [dnom]
  imm_zero/1 -> RelationalOperator/2
  RelationalOperator/1 -> Switch5/2
  [nume] -> Divide/1
  [dnom] -> Divide/2
  [nume] -> Switch5/3
  [dnom] -> RelationalOperator/1
  [xdat] -> Selector2/1
  [cons0] -> Selector2/2
  Selector2/1 -> Relational_Operator/2
  [ydat2] -> Sum2/1
  [ydat1] -> Sum2/2, RelationalOperator2/2
  [xdat] -> Selector6/1
  [xlen] -> Selector6/2
  [xdat] -> DataTypeConversion1_2/1
  DataTypeConversion1_3/1 -> Sum_of_Elements1/1
  Relational_Operator3/1 -> DataTypeConversion1_3/1
  DataTypeConversion1_2/1 -> Relational_Operator3/1, Relational_Operator3/2
  [ydat] -> Selector5/1
  [ylen] -> Selector5/2
  Divide/1 -> Switch5/1
  RelationalOperator2/1 -> Switch3/2
  [ydat2] -> Sum5/1, RelationalOperator2/1
  [ydat1] -> Sum5/2
  Sum5/1 -> Product2/2
  Switch5/1 -> [divide_lup1]
  [divide_lup1] -> Product2/1
  Sum3/1 -> Switch3/1
  [ydat1] -> Sum7/1
  Product1/1 -> Sum7/2
  [divide_lup1] -> Product1/1
  Sum7/1 -> Switch3/3
  Sum2/1 -> Product1/2
  Switch3/1 -> Switch1/3
  Relational_Operator/1 -> Switch/2
  [xlen] -> Sat_blk/1(Threshold_Upper)
  Sum4/1 -> Mux3/2
  Mux3/1 -> Sat_blk/2(In)
  Sat_blk/1(Out) -> [temp2], tempx1/2
  Constant1/1 -> [cons1]
  Switch2/1 -> Terminator/1
  [xdat] -> Switch2/1
  [ydat] -> Switch2/3
  Constant2/1 -> UnitDelay/1
  UnitDelay/1 -> Switch2/2
  Sum_of_Elements1/1 -> Sum8/1
  [cons1] -> Sum8/2
  Sum8/1 -> [xlen], [ylen]
  SumofElements2/1 -> Sum9/1
  Sum9/1 -> Mux3/1, Sum4/1

== system Renault_Library/Complex blocks/Lookup Tables/RSA_Sine_v01_03 ==
  u : Inport
      settings: AttributesFormatString=Type : %<OutDataTypeStr>
  Amp1 : Sum  Inputs=-+  OutDataTypeStr=Inherit: Inherit via back propagation  RndMeth=Zero  SaturateOnIntegerOverflow=on  InputSameDT=on  LockScale=on
      settings: AttributesFormatString=SAT = %<SaturateOnIntegerOverflow>
  Amp50 : Sum  Inputs=+-  OutDataTypeStr=Inherit: Inherit via back propagation  RndMeth=Zero  SaturateOnIntegerOverflow=on  InputSameDT=on  LockScale=on
      settings: AttributesFormatString=SAT = %<SaturateOnIntegerOverflow>
  Constant2 : Constant  Value=0.5  OutDataTypeStr=Inherit: Inherit via back propagation  SampleTime=-1
  Constant3 : Constant  Value=0.25  OutDataTypeStr=Inherit: Inherit via back propagation  SampleTime=-1
  Constant6 : Constant  Value=-1  OutDataTypeStr=int8  SampleTime=-1
  DataTypeConversion : DataTypeConversion  OutDataTypeStr=fixdt(0, OutputWordLength, OutputWordLength)
  Look_Up_Table : Lookup  InputValues=k  Table=b  LookUpMeth=Interpolation-Use End Values  OutDataTypeStr=fixdt(1, OutputWordLength, OutputFracLength)  RndMeth=Zero  SaturateOnIntegerOverflow=on
  Product : Product  OutDataTypeStr=Inherit: Inherit via back propagation  SaturateOnIntegerOverflow=on  RndMeth=Zero
      settings: AttributesFormatString=SAT = %<SaturateOnIntegerOverflow>
  Relational_Operator1 : RelationalOperator  Operator=<=  OutDataTypeStr=boolean  RndMeth=Nearest  InputSameDT=on
  Relational_Operator3 : RelationalOperator  Operator=<=  OutDataTypeStr=boolean  RndMeth=Nearest  InputSameDT=on
  Switch1 : Switch  Criteria=u2 ~= 0  RndMeth=Zero  InputSameDT=on
      settings: AttributesFormatString=Criteria=%<Criteria>
  Switch2 : Switch  Criteria=u2 ~= 0  RndMeth=Zero  InputSameDT=on
      settings: AttributesFormatString=Criteria=%<Criteria>
  Switch4 : Switch  Criteria=u2 ~= 0  RndMeth=Zero  InputSameDT=on
      settings: AttributesFormatString=Criteria=%<Criteria>
  y : Outport
      settings: AttributesFormatString=Type : %<OutDataTypeStr>\nInit :%<InitialOutput>
  wires:
  Relational_Operator1/1 -> [lespoint5], Switch1/2
  u/1 -> DataTypeConversion/1
  Amp50/1 -> Switch1/3
  Switch1/1 -> Relational_Operator3/1, Amp1/1, Switch4/1
  Constant3/1 -> Relational_Operator3/2
  Relational_Operator3/1 -> Switch4/2
  Amp1/1 -> Switch4/3
  [lespoint5] -> Switch2/2
  Switch2/1 -> y/1
  Constant2/1 -> Relational_Operator1/2, Amp50/2, Amp1/2
  Switch4/1 -> Look_Up_Table/1
  Look_Up_Table/1 -> Switch2/1, Product/1
  DataTypeConversion/1 -> Relational_Operator1/1, Switch1/1, [IniIO]
  [IniIO] -> Amp50/1
  Product/1 -> Switch2/3
  Constant6/1 -> Product/2

== system Renault_Library/Complex blocks/Lookup Tables/RSA_Sine_v01_04 ==
  u : Inport
      settings: AttributesFormatString=Type : %<OutDataTypeStr>
  Amp1 : Sum  Inputs=-+  OutDataTypeStr=Inherit: Inherit via back propagation  RndMeth=Zero  InputSameDT=on  LockScale=on
      settings: AttributesFormatString=SAT = %<SaturateOnIntegerOverflow>
  Amp50 : Sum  Inputs=+-  OutDataTypeStr=Inherit: Inherit via back propagation  RndMeth=Zero  InputSameDT=on  LockScale=on
      settings: AttributesFormatString=SAT = %<SaturateOnIntegerOverflow>
  Constant2 : Constant  Value=CONS_P5  OutDataTypeStr=Inherit: Inherit via back propagation  SampleTime=-1
  Constant3 : Constant  Value=CONS_P25  OutDataTypeStr=Inherit: Inherit via back propagation  SampleTime=-1
  Constant6 : Constant  Value=CONS_M1  OutDataTypeStr=int8  SampleTime=-1
  DataTypeConversion : DataTypeConversion  OutDataTypeStr=fixdt(0, OutputWordLength, OutputWordLength)  RndMeth=Zero
  Look_Up_Table : Lookup  InputValues=k  Table=b  LookUpMeth=Interpolation-Use End Values  OutDataTypeStr=fixdt(1, OutputWordLength, OutputFracLength)  RndMeth=Zero  SaturateOnIntegerOverflow=on
  Product : Product  OutDataTypeStr=Inherit: Inherit via back propagation  RndMeth=Zero
      settings: AttributesFormatString=SAT = %<SaturateOnIntegerOverflow>
  Relational_Operator1 : RelationalOperator  Operator=<=  OutDataTypeStr=boolean  RndMeth=Nearest  InputSameDT=on
  Relational_Operator3 : RelationalOperator  Operator=<=  OutDataTypeStr=boolean  RndMeth=Nearest  InputSameDT=on
  Switch1 : Switch  Criteria=u2 ~= 0  RndMeth=Zero  InputSameDT=on
      settings: AttributesFormatString=Criteria=%<Criteria>
  Switch2 : Switch  Criteria=u2 ~= 0  RndMeth=Zero  InputSameDT=on
      settings: AttributesFormatString=Criteria=%<Criteria>
  Switch4 : Switch  Criteria=u2 ~= 0  RndMeth=Zero  InputSameDT=on
      settings: AttributesFormatString=Criteria=%<Criteria>
  y : Outport
      settings: AttributesFormatString=Type : %<OutDataTypeStr>\nInit :%<InitialOutput>
  wires:
  Relational_Operator1/1 -> [lespoint5], Switch1/2
  u/1 -> DataTypeConversion/1
  Amp50/1 -> Switch1/3
  Switch1/1 -> Relational_Operator3/1, Amp1/1, Switch4/1
  Constant3/1 -> Relational_Operator3/2
  Relational_Operator3/1 -> Switch4/2
  Amp1/1 -> Switch4/3
  [lespoint5] -> Switch2/2
  Switch2/1 -> y/1
  Constant2/1 -> Relational_Operator1/2, Amp50/2, Amp1/2
  Switch4/1 -> Look_Up_Table/1
  Look_Up_Table/1 -> Switch2/1, Product/1
  DataTypeConversion/1 -> Relational_Operator1/1, Switch1/1, [IniIO]
  [IniIO] -> Amp50/1
  Product/1 -> Switch2/3
  Constant6/1 -> Product/2

== system Renault_Library/Complex blocks/Math Operations/RSA_ADivB ==
  A : Inport
      settings: AttributesFormatString=Type : %<OutDataTypeStr>
  B : Inport  Port=2
      settings: AttributesFormatString=Type : %<OutDataTypeStr>
  Constant : Constant  Value=eps  OutDataTypeStr=Inherit: Inherit via back propagation  SampleTime=-1
  Divide : Product  Inputs=*/  OutDataTypeStr=Inherit: Inherit via back propagation  RndMeth=Zero
      settings: AttributesFormatString=SAT = %<SaturateOnIntegerOverflow>
  RSA_CompareToZero : $bdroot/Complex blocks/Logic & Bit Operations/RSA_CompareToZero  Tag=[LIB]  relop===  LogicOutDataTypeMode=boolean
      settings: AttributesFormatString=%<MaskType> : Ver : 01.03\nOperator: %<relop>\nOutput data type mode: %<LogicOutDataTypeMode>\nEnable zero-crossing detection %<ZeroCross>
  Switch : Switch  Criteria=u2 ~= 0  RndMeth=Zero  InputSameDT=on
      settings: AttributesFormatString=Criteria=%<Criteria>
  AdivB : Outport
      settings: AttributesFormatString=Type : %<OutDataTypeStr>\nInit :%<InitialOutput>
  wires:
  B/1 -> RSA_CompareToZero/1, Switch/3
  Divide/1 -> AdivB/1
  Switch/1 -> Divide/2
  Constant/1 -> Switch/1
  RSA_CompareToZero/1 -> Switch/2
  A/1 -> Divide/1

== system Renault_Library/Complex blocks/Math Operations/RSA_DivisionByZero ==
  note: Division by "0"
      | with enabled subsystem
  note: Corresponding C code:
      |
      | if(den~=0) {
      |   ans = num/den;
      | }
      | else {
      |   ans = ans_of_0div;
      | }
  den : Inport  Tag=AM,In,0,1,Name,C;CMP,Port,PortWidth,SampleTime;
      settings: AttributesFormatString=Type : %<OutDataTypeStr>
  num : Inport  Tag=AM,In,0,1,Name,C;CMP,Port,PortWidth,SampleTime;  Port=2
      settings: AttributesFormatString=Type : %<OutDataTypeStr>
  ans_of_0div : Inport  Tag=AM,In,0,1,Name,C;CMP,Port,PortWidth,SampleTime;  Port=3
      settings: AttributesFormatString=Type : %<OutDataTypeStr>
  Perform_Division : Subsystem
  RelationalOperator : RelationalOperator  Tag=AM,Std,2,1;CMP,Operator;  Operator=~=  OutDataTypeStr=boolean  RndMeth=Nearest  InputSameDT=on
  Switch : Switch  Tag=GEN,Threshold;AM,Switch,2,1;CMP,Threshold;  Criteria=u2 ~= 0  RndMeth=Zero  SaturateOnIntegerOverflow=on  InputSameDT=on
      settings: AttributesFormatString=Criteria=%<Criteria>
  imm_zero : Constant  Tag=AM,Constant,0,1,Value;CMP,Value;  Value=DIVBY0  OutDataTypeStr=Inherit: Inherit via back propagation  SampleTime=-1
  ans : Outport  Tag=AM,Out,1,0,Name,NC;CMP,Port,OutputWhenDisabled,InitialOutput;
      settings: AttributesFormatString=Type : %<OutDataTypeStr>\nInit :%<InitialOutput>
  wires:
  [Enable_Division] -> Perform_Division/Enable  "Enable_Division"
  num/1 -> Perform_Division/1(num)
  den/1 -> Perform_Division/2(den), RelationalOperator/1
  Switch/1 -> ans/1
  ans_of_0div/1 -> Switch/3
  imm_zero/1 -> RelationalOperator/2
  RelationalOperator/1 -> [Enable_Division], Switch/2
  Perform_Division/1(ans) -> Switch/1
